simulink library "lib_all"  (Simulink 7.6)
settings: Solver=ode45  SolverMode=SingleTasking  StartTime=0.0  StopTime=10.0  RelTol=1e-3
values from neighbouring files (symbols this model uses):
  map_lib_03 = [6.8679 7.0159 7.4571 8.1849 9.1892 10.456 11.97 13.71 15.655 17.781 20.063 22.474 ... (803 elements, 73x11)]    [scr_simdata.mat]    (also in scr_simdata_all.mat)
  map_lib_06 = [2.5784 2.6727 2.9588 3.4453 4.1444 5.068 6.226 7.6242 9.2634 11.139 13.24 15.552 ... (803 elements, 73x11)]    [scr_simdata.mat]    (also in scr_simdata_all.mat)
  map_lib_09 = [0.0025784 0.0026727 0.0029588 0.0034453 0.0041444 0.005068 0.006226 0.0076242 0.0092634 0.011139 0.01324 0.015552 ... (803 elements, 73x11)]    [scr_simdata.mat]    (also in scr_simdata_all.mat)
  map_lib_10 = [2.74 2.75 2.76 2.77 2.78 2.79 2.8 2.81 2.82 2.83 2.84 2.85 ... (81 elements, 1x81)]    [scr_simdata.mat]    (also in scr_simdata_all.mat)
  map_lib_11 = [1.1983 1.2027 1.207 1.2113 1.2156 1.2198 1.2241 1.2283 1.2326 1.2368 1.241 1.2451 ... (81 elements, 1x81)]    [scr_simdata.mat]    (also in scr_simdata_all.mat)
  map_lib_12 = [2.74 2.75 2.76 2.77 2.78 2.79 2.8 2.81 2.82 2.83 2.84 2.85 ... (81 elements, 1x81)]    [scr_simdata.mat]    (also in scr_simdata_all.mat)
  map_lib_13 = [1.74 1.75 1.76 1.77 1.78 1.79 1.8 1.81 1.82 1.83 1.84 1.85 ... (81 elements, 1x81)]    [scr_simdata.mat]    (also in scr_simdata_all.mat)
  map_lib_16 = [0.00048828125 1.6159 1.8962 2.0821 2.2251 2.3426 2.4433 2.5318 2.611 2.683 2.749 2.8101 ... (1419 elements, 129x11)]    [scr_simdata.mat]    (also in scr_simdata_all.mat)
  map_mr2_01 = [0 800 1600 2400 3200 4000]    [scr_simdata.mat]    (also in scr_simdata_all.mat)
  map_mr2_02 = [1.32 1.32 1.33 1.34 1.36 1.38]    [scr_simdata.mat]    (also in scr_simdata_all.mat)
  map_mr2_03 = [0 800 1600 2400 3200 4000]    [scr_simdata.mat]    (also in scr_simdata_all.mat)
  map_mr2_04 = [1.32 1.32 1.33 1.34 1.36 1.38]    [scr_simdata.mat]    (also in scr_simdata_all.mat)
  map_mr2_05 = [0 800 1600 2400 3200 4000]    [scr_simdata.mat]    (also in scr_simdata_all.mat)
  map_mr2_06 = [1.32 1.32 1.33 1.34 1.36 1.38]    [scr_simdata.mat]    (also in scr_simdata_all.mat)
  Parameter1 = 6    [scr_simdata.mat]    (also in scr_simdata_all.mat)
  Parameter101 = 30    [scr_simdata.mat]    (also in scr_simdata_all.mat)
  Parameter102 = 12    [scr_simdata.mat]    (also in scr_simdata_all.mat)
  Parameter103 = -240    [scr_simdata.mat]    (also in scr_simdata_all.mat)
  Parameter108 = 48    [scr_simdata.mat]    (also in scr_simdata_all.mat)
  Parameter120 = 16    [scr_simdata.mat]    (also in scr_simdata_all.mat)
  Parameter121 = 87    [scr_simdata.mat]    (also in scr_simdata_all.mat)
  Parameter123 = 78    [scr_simdata.mat]    (also in scr_simdata_all.mat)
  Parameter124 = 60    [scr_simdata.mat]    (also in scr_simdata_all.mat)
  Parameter125 = -480    [scr_simdata.mat]    (also in scr_simdata_all.mat)
  Parameter130 = 96    [scr_simdata.mat]    (also in scr_simdata_all.mat)
  Parameter131 = 3    [scr_simdata.mat]    (also in scr_simdata_all.mat)
  Parameter144 = 3    [scr_simdata.mat]    (also in scr_simdata_all.mat)
  Parameter18 = 128    [scr_simdata.mat]    (also in scr_simdata_all.mat)
  Parameter19 = 16    [scr_simdata.mat]    (also in scr_simdata_all.mat)
  Parameter2 = 12    [scr_simdata.mat]    (also in scr_simdata_all.mat)
  Parameter20 = 7    [scr_simdata.mat]    (also in scr_simdata_all.mat)
  Parameter21 = 4    [scr_simdata.mat]    (also in scr_simdata_all.mat)
  Parameter3 = 18    [scr_simdata.mat]    (also in scr_simdata_all.mat)
  Parameter32 = 1    [scr_simdata.mat]    (also in scr_simdata_all.mat)
  Parameter34 = 24    [scr_simdata.mat]    (also in scr_simdata_all.mat)
  Parameter4 = 138    [scr_simdata.mat]    (also in scr_simdata_all.mat)
  Parameter42 = 48    [scr_simdata.mat]    (also in scr_simdata_all.mat)
  Parameter5 = 135    [scr_simdata.mat]    (also in scr_simdata_all.mat)
  Parameter55 = 0    [scr_simdata.mat]    (also in scr_simdata_all.mat)
  Parameter56 = 0    [scr_simdata.mat]    (also in scr_simdata_all.mat)
  Parameter57 = 1    [scr_simdata.mat]    (also in scr_simdata_all.mat)
  Parameter58 = 1    [scr_simdata.mat]    (also in scr_simdata_all.mat)
  Parameter59 = 2    [scr_simdata.mat]    (also in scr_simdata_all.mat)
  Parameter6 = 132    [scr_simdata.mat]    (also in scr_simdata_all.mat)
  Parameter60 = 2    [scr_simdata.mat]    (also in scr_simdata_all.mat)
  Parameter63 = 144    [scr_simdata.mat]    (also in scr_simdata_all.mat)
  Parameter7 = 126    [scr_simdata.mat]    (also in scr_simdata_all.mat)
  Parameter76 = 64    [scr_simdata.mat]    (also in scr_simdata_all.mat)
  Parameter77 = 135    [scr_simdata.mat]    (also in scr_simdata_all.mat)
  Parameter79 = 126    [scr_simdata.mat]    (also in scr_simdata_all.mat)
  Parameter80 = 108    [scr_simdata.mat]    (also in scr_simdata_all.mat)
  Parameter81 = 0    [scr_simdata.mat]    (also in scr_simdata_all.mat)
  Parameter86 = 0    [scr_simdata.mat]    (also in scr_simdata_all.mat)
  Parameter98 = 112    [scr_simdata.mat]    (also in scr_simdata_all.mat)
  Parameter99 = 39    [scr_simdata.mat]    (also in scr_simdata_all.mat)
undefined symbols (not defined in the file or in its folder): cylvolkp_hih_map2h, cylvolkp_map2h, pk1k_map2h
libraries: simulink x2
(part 11 of 36)

== system lib_all/SubSystem1196/SubSystem1273/SubSystem1328/SubSystem1330 ==
  note: Text255
  Block3578 : Inport
  Block3579 : Inport  Port=2
  Block3580 : Inport  Port=3
  Block3581 : Constant  Value=0  OutDataTypeStr=double  SampleTime=-1
  Block3582 : Constant  Value=720  OutDataTypeStr=double  SampleTime=-1
  Block3583 : Gain  Gain=5  SaturateOnIntegerOverflow=on  OutDataTypeStr=Inherit: Same as input  ParamDataTypeStr=Inherit: Same as input
      settings: AttributesFormatString=Attribute703
  Block3584 : Sum  Inputs=+-  OutDataTypeStr=double  RndMeth=Zero  SaturateOnIntegerOverflow=on  InputSameDT=on
  SubSystem1331 : Subsystem
      settings: RTWFcnNameOpts=Use subsystem name
  SubSystem1336 : Subsystem
      settings: RTWSystemCode=Inline
  Block3610 : Outport
  wires:
  Block3583/1 -> Block3584/1
  SubSystem1331/1(Block3603) -> Block3583/1
  Block3578/1 -> SubSystem1331/1(Block3585)
  Block3579/1 -> SubSystem1331/2(Block3586)
  Block3580/1 -> Block3584/2
  Block3584/1 -> SubSystem1336/2(Block3605)
  Block3582/1 -> SubSystem1336/1(Block3604)
  Block3581/1 -> SubSystem1336/3(Block3606)
  SubSystem1336/1(Block3609) -> Block3610/1

== system lib_all/SubSystem1196/SubSystem1273/SubSystem1328/SubSystem1338 ==
  note: Text256
  Block3611 : Inport
  Block3612 : Inport  Port=2
  Block3613 : Constant  Value=360  OutDataTypeStr=double  SampleTime=-1
  Block3614 : Constant  Value=360  OutDataTypeStr=double  SampleTime=-1
  Block3615 : Sum  Inputs=+-  OutDataTypeStr=double  RndMeth=Zero  SaturateOnIntegerOverflow=on  InputSameDT=on
  Block3616 : Switch  Threshold=0.5  RndMeth=Zero  SaturateOnIntegerOverflow=on  InputSameDT=on
  Block3617 : Lookup2D  RowIndex=map_lib_08  ColumnIndex=map_lib_07  Table=map_lib_09  SaturateOnIntegerOverflow=on  InputSameDT=on
      settings: AttributesFormatString=<%<OutputValues>>\nAttribute712
  SubSystem1339 : Subsystem
  Block3618 : Outport
  wires:
  Block3612/1 -> SubSystem1339/1(In1), Block3616/3, Block3615/1
  Block3614/1 -> SubSystem1339/2(In2)
  SubSystem1339/1(Out1) -> Block3616/2
  Block3615/1 -> Block3616/1
  Block3613/1 -> Block3615/2
  Block3617/1 -> Block3618/1
  Block3611/1 -> Block3617/2
  Block3616/1 -> Block3617/1  "SigLabel117"

== system lib_all/SubSystem1196/SubSystem1340/SubSystem1342/SubSystem1343 ==
  Block3632 : Inport
  Block3633 : Inport  Port=2
  Block3634 : Constant  Value=Parameter32  OutDataTypeStr=double  SampleTime=-1
  Block3635 : Constant  Value=2  OutDataTypeStr=double  SampleTime=-1
  Block3636 : Constant  Value=Parameter42  OutDataTypeStr=double  SampleTime=-1
  Block3637 : Constant  Value=0  OutDataTypeStr=double  SampleTime=-1
  Block3638 : Constant  Value=72  OutDataTypeStr=double  SampleTime=-1
  Block3639 : Constant  Value=Parameter34  OutDataTypeStr=double  SampleTime=-1
  Block3640 : Logic  Operator=OR
  Block3641 : Sum  Inputs=+-  OutDataTypeStr=double  RndMeth=Zero  SaturateOnIntegerOverflow=on  InputSameDT=on
  Block3642 : Switch  Threshold=0.5  RndMeth=Zero  SaturateOnIntegerOverflow=on  InputSameDT=on
  Block3643 : Switch  Threshold=0.5  RndMeth=Zero  SaturateOnIntegerOverflow=on  InputSameDT=on
  SubSystem1344 : Subsystem
  SubSystem1345 : Subsystem
  SubSystem1346 : Subsystem
      settings: RTWSystemCode=Inline
  Block3650 : Outport
  wires:
  Block3643/1 -> Block3650/1
  SubSystem1346/1(Block3649) -> Block3643/1
  Block3638/1 -> SubSystem1346/1(Block3644)
  Block3637/1 -> SubSystem1346/3(Block3646)
  Block3641/1 -> SubSystem1346/2(Block3645)
  Block3632/1 -> Block3643/3, Block3641/1
  Block3633/1 -> SubSystem1344/2(In2), SubSystem1345/1(In1)
  Block3634/1 -> SubSystem1344/1(In1)
  Block3635/1 -> SubSystem1345/2(In2)
  SubSystem1344/1(Out1) -> Block3640/1, Block3642/2
  SubSystem1345/1(Out1) -> Block3640/2
  Block3640/1 -> Block3643/2
  Block3636/1 -> Block3642/1
  Block3639/1 -> Block3642/3
  Block3642/1 -> Block3641/2

== system lib_all/SubSystem1196/SubSystem1340/SubSystem1342/SubSystem1348 ==
  Block3651 : Inport
  Block3652 : Inport  Port=2
  Block3653 : Inport  Port=3
  Block3654 : Sum  OutDataTypeStr=double  RndMeth=Zero  SaturateOnIntegerOverflow=on  InputSameDT=on
  Block3655 : Switch  Threshold=0.5  RndMeth=Zero  SaturateOnIntegerOverflow=on  InputSameDT=on
  SubSystem1349 : Subsystem
  Block3656 : Outport
  wires:
  Block3652/1 -> SubSystem1349/1(In1), Block3655/3, Block3654/2
  Block3653/1 -> SubSystem1349/2(In2)
  SubSystem1349/1(Out1) -> Block3655/2
  Block3654/1 -> Block3655/1
  Block3655/1 -> Block3656/1
  Block3651/1 -> Block3654/1

== system lib_all/SubSystem1196/SubSystem1340/SubSystem1350/SubSystem1351 ==
  note: ***編集禁止***
  In1 : Inport
  In2 : Inport  Port=2
  RO1 : RelationalOperator  Operator=>  InputSameDT=on
  single1 : DataTypeConversion  OutDataTypeStr=single  SaturateOnIntegerOverflow=on  RndMeth=Zero
  single2 : DataTypeConversion  OutDataTypeStr=single  SaturateOnIntegerOverflow=on  RndMeth=Zero
  Out1 : Outport
  wires:
  single2/1 -> RO1/2
  single1/1 -> RO1/1
  In1/1 -> single1/1
  In2/1 -> single2/1
  RO1/1 -> Out1/1

== system lib_all/SubSystem1196/SubSystem1352/SubSystem1354/SubSystem1355 ==
  Block3678 : Inport
  Block3679 : Inport  Port=2
  Block3680 : Constant  Value=Parameter32  OutDataTypeStr=double  SampleTime=-1
  Block3681 : Constant  Value=2  OutDataTypeStr=double  SampleTime=-1
  Block3682 : Constant  Value=Parameter42  OutDataTypeStr=double  SampleTime=-1
  Block3683 : Constant  Value=0  OutDataTypeStr=double  SampleTime=-1
  Block3684 : Constant  Value=72  OutDataTypeStr=double  SampleTime=-1
  Block3685 : Constant  Value=Parameter34  OutDataTypeStr=double  SampleTime=-1
  Block3686 : Logic  Operator=OR
  Block3687 : Sum  Inputs=+-  OutDataTypeStr=double  RndMeth=Zero  SaturateOnIntegerOverflow=on  InputSameDT=on
  Block3688 : Switch  Threshold=0.5  RndMeth=Zero  SaturateOnIntegerOverflow=on  InputSameDT=on
  Block3689 : Switch  Threshold=0.5  RndMeth=Zero  SaturateOnIntegerOverflow=on  InputSameDT=on
  SubSystem1356 : Subsystem
  SubSystem1357 : Subsystem
  SubSystem1358 : Subsystem
      settings: RTWSystemCode=Inline
  Block3696 : Outport
  wires:
  Block3689/1 -> Block3696/1
  SubSystem1358/1(Block3695) -> Block3689/1
  Block3684/1 -> SubSystem1358/1(Block3690)
  Block3683/1 -> SubSystem1358/3(Block3692)
  Block3687/1 -> SubSystem1358/2(Block3691)
  Block3678/1 -> Block3689/3, Block3687/1
  Block3679/1 -> SubSystem1356/2(In2), SubSystem1357/1(In1)
  Block3680/1 -> SubSystem1356/1(In1)
  Block3681/1 -> SubSystem1357/2(In2)
  SubSystem1356/1(Out1) -> Block3686/1, Block3688/2
  SubSystem1357/1(Out1) -> Block3686/2
  Block3686/1 -> Block3689/2
  Block3682/1 -> Block3688/1
  Block3685/1 -> Block3688/3
  Block3688/1 -> Block3687/2

== system lib_all/SubSystem1196/SubSystem1352/SubSystem1354/SubSystem1360 ==
  Block3697 : Inport
  Block3698 : Inport  Port=2
  Block3699 : Inport  Port=3
  Block3700 : Sum  OutDataTypeStr=double  RndMeth=Zero  SaturateOnIntegerOverflow=on  InputSameDT=on
  Block3701 : Switch  Threshold=0.5  RndMeth=Zero  SaturateOnIntegerOverflow=on  InputSameDT=on
  SubSystem1361 : Subsystem
  Block3702 : Outport
  wires:
  Block3698/1 -> SubSystem1361/1(In1), Block3701/3, Block3700/2
  Block3699/1 -> SubSystem1361/2(In2)
  SubSystem1361/1(Out1) -> Block3701/2
  Block3700/1 -> Block3701/1
  Block3701/1 -> Block3702/1
  Block3697/1 -> Block3700/1

== system lib_all/SubSystem1196/SubSystem1352/SubSystem1362/SubSystem1363 ==
  note: ***編集禁止***
  In1 : Inport
  In2 : Inport  Port=2
  RO1 : RelationalOperator  Operator=>  InputSameDT=on
  single1 : DataTypeConversion  OutDataTypeStr=single  SaturateOnIntegerOverflow=on  RndMeth=Zero
  single2 : DataTypeConversion  OutDataTypeStr=single  SaturateOnIntegerOverflow=on  RndMeth=Zero
  Out1 : Outport
  wires:
  single2/1 -> RO1/2
  single1/1 -> RO1/1
  In1/1 -> single1/1
  In2/1 -> single2/1
  RO1/1 -> Out1/1

== system lib_all/SubSystem1364/SubSystem1366/SubSystem1369/SubSystem1370 ==
  note: ***編集禁止***
  mult : Inport
  div : Inport  Port=2
  init : Inport  Port=3
  Product : Subsystem  TreatAsAtomicUnit=on
      settings: RTWSystemCode=Inline
  Out : Outport
  wires:
  init/1 -> Product/3(init)
  div/1 -> Product/2(div)
  mult/1 -> Product/1(mult)
  Product/1(Out) -> Out/1

== system lib_all/SubSystem1364/SubSystem1366/SubSystem1369/SubSystem1371 ==
  note: ***編集禁止***
  element : Inport
  Inside_DSR : DataStoreRead  DataStoreName=DSM95
  Selector : Selector  IndexOptions=Index vector (port)  Indices=[1 3]  OutputSizes=1
  Output : Outport
  wires:
  Inside_DSR/1 -> Selector/1
  element/1 -> Selector/2
  Selector/1 -> Output/1

== system lib_all/SubSystem1364/SubSystem1366/SubSystem1369/SubSystem1372 ==
  Block3761 : Inport
  Block3762 : Inport  Port=2
  Block3763 : Inport  Port=3
  Block3764 : Sum  OutDataTypeStr=double  RndMeth=Zero  SaturateOnIntegerOverflow=on  InputSameDT=on
  Block3765 : Sum  Inputs=+-  OutDataTypeStr=double  RndMeth=Zero  SaturateOnIntegerOverflow=on  InputSameDT=on
  Block3766 : Switch  Threshold=0.5  RndMeth=Zero  SaturateOnIntegerOverflow=on  InputSameDT=on
  Block3767 : Switch  Threshold=0.5  RndMeth=Zero  SaturateOnIntegerOverflow=on  InputSameDT=on
  SubSystem1373 : Subsystem
  SubSystem1374 : Subsystem
  Block3768 : Outport
  wires:
  Block3765/1 -> Block3766/1
  Block3766/1 -> Block3768/1
  Block3764/1 -> Block3767/1
  Block3767/1 -> Block3766/3
  SubSystem1374/1(Out1) -> Block3767/2
  Block3763/1 -> SubSystem1374/2(In2)
  SubSystem1373/1(Out1) -> Block3766/2
  Block3761/1 -> SubSystem1373/2(In2), Block3765/2, Block3764/1
  Block3762/1 -> Block3764/2, Block3767/3, SubSystem1374/1(In1), Block3765/1, SubSystem1373/1(In1)

== system lib_all/SubSystem1364/SubSystem1366/SubSystem1375/SubSystem1376 ==
  note: ***編集禁止***
  In1 : Inport
  In2 : Inport  Port=2
  LO1 : Logic
  RO1 : RelationalOperator  Operator=<  InputSameDT=on
  RO2 : RelationalOperator  Operator=~=  InputSameDT=on
  single1 : DataTypeConversion  OutDataTypeStr=single  SaturateOnIntegerOverflow=on  RndMeth=Zero
  single2 : DataTypeConversion  OutDataTypeStr=single  SaturateOnIntegerOverflow=on  RndMeth=Zero
  Out1 : Outport
  wires:
  single2/1 -> RO1/2, RO2/2
  single1/1 -> RO1/1, RO2/1
  In1/1 -> single1/1
  In2/1 -> single2/1
  RO1/1 -> LO1/1
  RO2/1 -> LO1/2
  LO1/1 -> Out1/1

== system lib_all/SubSystem1364/SubSystem1366/SubSystem1375/SubSystem1377 ==
  note: ***編集禁止***
  In1 : Inport
  In2 : Inport  Port=2
  RO1 : RelationalOperator  Operator===  InputSameDT=on
  single1 : DataTypeConversion  OutDataTypeStr=single  SaturateOnIntegerOverflow=on  RndMeth=Zero
  single2 : DataTypeConversion  OutDataTypeStr=single  SaturateOnIntegerOverflow=on  RndMeth=Zero
  Out1 : Outport
  wires:
  single2/1 -> RO1/2
  single1/1 -> RO1/1
  In1/1 -> single1/1
  In2/1 -> single2/1
  RO1/1 -> Out1/1

== system lib_all/SubSystem1364/SubSystem1366/SubSystem1375/SubSystem1378 ==
  note: ***編集禁止***
  In1 : Inport
  In2 : Inport  Port=2
  RO1 : RelationalOperator  Operator===  InputSameDT=on
  single1 : DataTypeConversion  OutDataTypeStr=single  SaturateOnIntegerOverflow=on  RndMeth=Zero
  single2 : DataTypeConversion  OutDataTypeStr=single  SaturateOnIntegerOverflow=on  RndMeth=Zero
  Out1 : Outport
  wires:
  single2/1 -> RO1/2
  single1/1 -> RO1/1
  In1/1 -> single1/1
  In2/1 -> single2/1
  RO1/1 -> Out1/1

== system lib_all/SubSystem1364/SubSystem1366/SubSystem1375/SubSystem1379 ==
  note: ***編集禁止***
  In1 : Inport
  In2 : Inport  Port=2
  RO1 : RelationalOperator  Operator===  InputSameDT=on
  single1 : DataTypeConversion  OutDataTypeStr=single  SaturateOnIntegerOverflow=on  RndMeth=Zero
  single2 : DataTypeConversion  OutDataTypeStr=single  SaturateOnIntegerOverflow=on  RndMeth=Zero
  Out1 : Outport
  wires:
  single2/1 -> RO1/2
  single1/1 -> RO1/1
  In1/1 -> single1/1
  In2/1 -> single2/1
  RO1/1 -> Out1/1

== system lib_all/SubSystem1364/SubSystem1366/SubSystem1375/SubSystem1380 ==
  Block3791 : Inport
  Block3792 : Inport  Port=2
  Block3793 : EnablePort
  Block3794 : Demux  Outputs=2
  Block3795 : Mux  Inputs=2
  SubSystem1381 : Subsystem
  SubSystem1385 : Subsystem
  Block3804 : Outport
  wires:
  Block3795/1 -> Block3804/1
  SubSystem1385/1(Block3803) -> Block3795/2
  SubSystem1381/1(Block3799) -> Block3795/1
  Block3794/2 -> SubSystem1385/2(Block3801)
  Block3794/1 -> SubSystem1381/2(Block3797)
  Block3792/1 -> SubSystem1381/1(Block3796), SubSystem1385/1(Block3800)
  Block3791/1 -> Block3794/1

== system lib_all/SubSystem1364/SubSystem1366/SubSystem1375/SubSystem1389 ==
  Block3805 : Inport
  Block3806 : Inport  Port=2
  Block3807 : EnablePort
  Block3808 : Demux  Outputs=2
  Block3809 : Mux  Inputs=2
  Block3810 : Sum  OutDataTypeStr=double  RndMeth=Zero  SaturateOnIntegerOverflow=on  InputSameDT=on
  SubSystem1390 : Subsystem  TreatAsAtomicUnit=on  mask(***ATC.DSRVEC***){bd_version=1.01.A, r0=DSM1, r1=-1, r2=, r3=, r4=, r5=, r6=, r7=, r8=, r9=, c0=, c1=, c2=, c3=, c4=, c5=, c6=, c7=, c8=, c9=}
      mask prompts: bd_version "Version"
      mask help: これはAutoCからのブロックです．編集禁止．
      settings: OpenFcn=warndlg('変更禁止!');
      mask Help: web(ui_blockhelpmng(gcb));
      mask Display: disp('ATC.DSRVEC')
  SubSystem1391 : Subsystem
  SubSystem1395 : Subsystem
  Block3819 : Outport
  wires:
  Block3806/1 -> SubSystem1390/1(element), SubSystem1391/1(Block3811), SubSystem1395/1(Block3815)
  Block3809/1 -> Block3810/2
  SubSystem1390/1(Output) -> Block3810/1
  Block3810/1 -> Block3819/1
  SubSystem1395/1(Block3818) -> Block3809/2
  SubSystem1391/1(Block3814) -> Block3809/1
  Block3808/2 -> SubSystem1395/2(Block3816)
  Block3808/1 -> SubSystem1391/2(Block3812)
  Block3805/1 -> Block3808/1

== system lib_all/SubSystem1364/SubSystem1366/SubSystem1375/SubSystem1399 ==
  Block3820 : Inport
  Block3821 : Inport  Port=2
  Block3822 : EnablePort
  Block3823 : Demux  Outputs=2
  Block3824 : Mux  Inputs=2
  Block3825 : Sum  OutDataTypeStr=double  RndMeth=Zero  SaturateOnIntegerOverflow=on  InputSameDT=on
  SubSystem1400 : Subsystem  TreatAsAtomicUnit=on  mask(***ATC.DSRVEC***){bd_version=1.01.A, r0=DSM5, r1=-1, r2=, r3=, r4=, r5=, r6=, r7=, r8=, r9=, c0=, c1=, c2=, c3=, c4=, c5=, c6=, c7=, c8=, c9=}
      mask prompts: bd_version "Version"
      mask help: これはAutoCからのブロックです．編集禁止．
      settings: OpenFcn=warndlg('変更禁止!');
      mask Help: web(ui_blockhelpmng(gcb));
      mask Display: disp('ATC.DSRVEC')
  SubSystem1401 : Subsystem
  SubSystem1405 : Subsystem
  Block3834 : Outport
  wires:
  Block3821/1 -> SubSystem1400/1(element), SubSystem1401/1(Block3826), SubSystem1405/1(Block3830)
  Block3824/1 -> Block3825/2
  SubSystem1400/1(Output) -> Block3825/1
  Block3825/1 -> Block3834/1
  SubSystem1405/1(Block3833) -> Block3824/2
  SubSystem1401/1(Block3829) -> Block3824/1
  Block3823/2 -> SubSystem1405/2(Block3831)
  Block3823/1 -> SubSystem1401/2(Block3827)
  Block3820/1 -> Block3823/1

== system lib_all/SubSystem1364/SubSystem1366/SubSystem1375/SubSystem1409 ==
  Block3835 : Inport
  Block3836 : Inport  Port=2
  Block3837 : EnablePort
  Block3838 : Demux  Outputs=2
  Block3839 : Mux  Inputs=2
  Block3840 : Sum  OutDataTypeStr=double  RndMeth=Zero  SaturateOnIntegerOverflow=on  InputSameDT=on
  SubSystem1410 : Subsystem  TreatAsAtomicUnit=on  mask(***ATC.DSRVEC***){bd_version=1.01.A, r0=DSM3, r1=-1, r2=, r3=, r4=, r5=, r6=, r7=, r8=, r9=, c0=, c1=, c2=, c3=, c4=, c5=, c6=, c7=, c8=, c9=}
      mask prompts: bd_version "Version"
      mask help: これはAutoCからのブロックです．編集禁止．
      settings: OpenFcn=warndlg('変更禁止!');
      mask Help: web(ui_blockhelpmng(gcb));
      mask Display: disp('ATC.DSRVEC')
  SubSystem1411 : Subsystem
  SubSystem1415 : Subsystem
  Block3849 : Outport
  wires:
  Block3836/1 -> SubSystem1410/1(element), SubSystem1411/1(Block3841), SubSystem1415/1(Block3845)
  Block3835/1 -> Block3838/1
  Block3838/1 -> SubSystem1411/2(Block3842)
  Block3838/2 -> SubSystem1415/2(Block3846)
  SubSystem1411/1(Block3844) -> Block3839/1
  SubSystem1415/1(Block3848) -> Block3839/2
  Block3840/1 -> Block3849/1
  SubSystem1410/1(Output) -> Block3840/1
  Block3839/1 -> Block3840/2

== system lib_all/SubSystem1364/SubSystem1419/SubSystem1422/SubSystem1423 ==
  note: ***編集禁止***
  mult : Inport
  div : Inport  Port=2
  init : Inport  Port=3
  Product : Subsystem  TreatAsAtomicUnit=on
      settings: RTWSystemCode=Inline
  Out : Outport
  wires:
  init/1 -> Product/3(init)
  div/1 -> Product/2(div)
  mult/1 -> Product/1(mult)
  Product/1(Out) -> Out/1

== system lib_all/SubSystem1364/SubSystem1419/SubSystem1422/SubSystem1424 ==
  note: ***編集禁止***
  element : Inport
  Inside_DSR : DataStoreRead  DataStoreName=DSM95
  Selector : Selector  IndexOptions=Index vector (port)  Indices=[1 3]  OutputSizes=1
  Output : Outport
  wires:
  Inside_DSR/1 -> Selector/1
  element/1 -> Selector/2
  Selector/1 -> Output/1

== system lib_all/SubSystem1364/SubSystem1419/SubSystem1422/SubSystem1425 ==
  Block3883 : Inport
  Block3884 : Inport  Port=2
  Block3885 : Inport  Port=3
  Block3886 : Sum  OutDataTypeStr=double  RndMeth=Zero  SaturateOnIntegerOverflow=on  InputSameDT=on
  Block3887 : Sum  Inputs=+-  OutDataTypeStr=double  RndMeth=Zero  SaturateOnIntegerOverflow=on  InputSameDT=on
  Block3888 : Switch  Threshold=0.5  RndMeth=Zero  SaturateOnIntegerOverflow=on  InputSameDT=on
  Block3889 : Switch  Threshold=0.5  RndMeth=Zero  SaturateOnIntegerOverflow=on  InputSameDT=on
  SubSystem1426 : Subsystem
  SubSystem1427 : Subsystem
  Block3890 : Outport
  wires:
  Block3887/1 -> Block3888/1
  Block3888/1 -> Block3890/1
  Block3886/1 -> Block3889/1
  Block3889/1 -> Block3888/3
  SubSystem1427/1(Out1) -> Block3889/2
  Block3885/1 -> SubSystem1427/2(In2)
  SubSystem1426/1(Out1) -> Block3888/2
  Block3883/1 -> SubSystem1426/2(In2), Block3887/2, Block3886/1
  Block3884/1 -> Block3886/2, Block3889/3, SubSystem1427/1(In1), Block3887/1, SubSystem1426/1(In1)

== system lib_all/SubSystem1364/SubSystem1419/SubSystem1428/SubSystem1429 ==
  note: ***編集禁止***
  In1 : Inport
  In2 : Inport  Port=2
  LO1 : Logic
  RO1 : RelationalOperator  Operator=<  InputSameDT=on
  RO2 : RelationalOperator  Operator=~=  InputSameDT=on
  single1 : DataTypeConversion  OutDataTypeStr=single  SaturateOnIntegerOverflow=on  RndMeth=Zero
  single2 : DataTypeConversion  OutDataTypeStr=single  SaturateOnIntegerOverflow=on  RndMeth=Zero
  Out1 : Outport
  wires:
  single2/1 -> RO1/2, RO2/2
  single1/1 -> RO1/1, RO2/1
  In1/1 -> single1/1
  In2/1 -> single2/1
  RO1/1 -> LO1/1
  RO2/1 -> LO1/2
  LO1/1 -> Out1/1

== system lib_all/SubSystem1364/SubSystem1419/SubSystem1428/SubSystem1430 ==
  note: ***編集禁止***
  In1 : Inport
  In2 : Inport  Port=2
  RO1 : RelationalOperator  Operator===  InputSameDT=on
  single1 : DataTypeConversion  OutDataTypeStr=single  SaturateOnIntegerOverflow=on  RndMeth=Zero
  single2 : DataTypeConversion  OutDataTypeStr=single  SaturateOnIntegerOverflow=on  RndMeth=Zero
  Out1 : Outport
  wires:
  single2/1 -> RO1/2
  single1/1 -> RO1/1
  In1/1 -> single1/1
  In2/1 -> single2/1
  RO1/1 -> Out1/1

== system lib_all/SubSystem1364/SubSystem1419/SubSystem1428/SubSystem1431 ==
  note: ***編集禁止***
  In1 : Inport
  In2 : Inport  Port=2
  RO1 : RelationalOperator  Operator===  InputSameDT=on
  single1 : DataTypeConversion  OutDataTypeStr=single  SaturateOnIntegerOverflow=on  RndMeth=Zero
  single2 : DataTypeConversion  OutDataTypeStr=single  SaturateOnIntegerOverflow=on  RndMeth=Zero
  Out1 : Outport
  wires:
  single2/1 -> RO1/2
  single1/1 -> RO1/1
  In1/1 -> single1/1
  In2/1 -> single2/1
  RO1/1 -> Out1/1

== system lib_all/SubSystem1364/SubSystem1419/SubSystem1428/SubSystem1432 ==
  note: ***編集禁止***
  In1 : Inport
  In2 : Inport  Port=2
  RO1 : RelationalOperator  Operator===  InputSameDT=on
  single1 : DataTypeConversion  OutDataTypeStr=single  SaturateOnIntegerOverflow=on  RndMeth=Zero
  single2 : DataTypeConversion  OutDataTypeStr=single  SaturateOnIntegerOverflow=on  RndMeth=Zero
  Out1 : Outport
  wires:
  single2/1 -> RO1/2
  single1/1 -> RO1/1
  In1/1 -> single1/1
  In2/1 -> single2/1
  RO1/1 -> Out1/1

== system lib_all/SubSystem1364/SubSystem1419/SubSystem1428/SubSystem1433 ==
  Block3913 : Inport
  Block3914 : Inport  Port=2
  Block3915 : EnablePort
  Block3916 : Demux  Outputs=2
  Block3917 : Mux  Inputs=2
  SubSystem1434 : Subsystem
  SubSystem1438 : Subsystem
  Block3926 : Outport
  wires:
  Block3917/1 -> Block3926/1
  SubSystem1438/1(Block3925) -> Block3917/2
  SubSystem1434/1(Block3921) -> Block3917/1
  Block3916/2 -> SubSystem1438/2(Block3923)
  Block3916/1 -> SubSystem1434/2(Block3919)
  Block3914/1 -> SubSystem1434/1(Block3918), SubSystem1438/1(Block3922)
  Block3913/1 -> Block3916/1

== system lib_all/SubSystem1364/SubSystem1419/SubSystem1428/SubSystem1442 ==
  Block3927 : Inport
  Block3928 : Inport  Port=2
  Block3929 : EnablePort
  Block3930 : Demux  Outputs=2
  Block3931 : Mux  Inputs=2
  Block3932 : Sum  OutDataTypeStr=double  RndMeth=Zero  SaturateOnIntegerOverflow=on  InputSameDT=on
  SubSystem1443 : Subsystem  TreatAsAtomicUnit=on  mask(***ATC.DSRVEC***){bd_version=1.01.A, r0=DSM1, r1=-1, r2=, r3=, r4=, r5=, r6=, r7=, r8=, r9=, c0=, c1=, c2=, c3=, c4=, c5=, c6=, c7=, c8=, c9=}
      mask prompts: bd_version "Version"
      mask help: これはAutoCからのブロックです．編集禁止．
      settings: OpenFcn=warndlg('変更禁止!');
      mask Help: web(ui_blockhelpmng(gcb));
      mask Display: disp('ATC.DSRVEC')
  SubSystem1444 : Subsystem
  SubSystem1448 : Subsystem
  Block3941 : Outport
  wires:
  Block3928/1 -> SubSystem1443/1(element), SubSystem1444/1(Block3933), SubSystem1448/1(Block3937)
  Block3931/1 -> Block3932/2
  SubSystem1443/1(Output) -> Block3932/1
  Block3932/1 -> Block3941/1
  SubSystem1448/1(Block3940) -> Block3931/2
  SubSystem1444/1(Block3936) -> Block3931/1
  Block3930/2 -> SubSystem1448/2(Block3938)
  Block3930/1 -> SubSystem1444/2(Block3934)
  Block3927/1 -> Block3930/1

== system lib_all/SubSystem1364/SubSystem1419/SubSystem1428/SubSystem1452 ==
  Block3942 : Inport
  Block3943 : Inport  Port=2
  Block3944 : EnablePort
  Block3945 : Demux  Outputs=2
  Block3946 : Mux  Inputs=2
  Block3947 : Sum  OutDataTypeStr=double  RndMeth=Zero  SaturateOnIntegerOverflow=on  InputSameDT=on
  SubSystem1453 : Subsystem  TreatAsAtomicUnit=on  mask(***ATC.DSRVEC***){bd_version=1.01.A, r0=DSM5, r1=-1, r2=, r3=, r4=, r5=, r6=, r7=, r8=, r9=, c0=, c1=, c2=, c3=, c4=, c5=, c6=, c7=, c8=, c9=}
      mask prompts: bd_version "Version"
      mask help: これはAutoCからのブロックです．編集禁止．
      settings: OpenFcn=warndlg('変更禁止!');
      mask Help: web(ui_blockhelpmng(gcb));
      mask Display: disp('ATC.DSRVEC')
  SubSystem1454 : Subsystem
  SubSystem1458 : Subsystem
  Block3956 : Outport
  wires:
  Block3943/1 -> SubSystem1453/1(element), SubSystem1454/1(Block3948), SubSystem1458/1(Block3952)
  Block3946/1 -> Block3947/2
  SubSystem1453/1(Output) -> Block3947/1
  Block3947/1 -> Block3956/1
  SubSystem1458/1(Block3955) -> Block3946/2
  SubSystem1454/1(Block3951) -> Block3946/1
  Block3945/2 -> SubSystem1458/2(Block3953)
  Block3945/1 -> SubSystem1454/2(Block3949)
  Block3942/1 -> Block3945/1

== system lib_all/SubSystem1364/SubSystem1419/SubSystem1428/SubSystem1462 ==
  Block3957 : Inport
  Block3958 : Inport  Port=2
  Block3959 : EnablePort
  Block3960 : Demux  Outputs=2
  Block3961 : Mux  Inputs=2
  Block3962 : Sum  OutDataTypeStr=double  RndMeth=Zero  SaturateOnIntegerOverflow=on  InputSameDT=on
  SubSystem1463 : Subsystem  TreatAsAtomicUnit=on  mask(***ATC.DSRVEC***){bd_version=1.01.A, r0=DSM3, r1=-1, r2=, r3=, r4=, r5=, r6=, r7=, r8=, r9=, c0=, c1=, c2=, c3=, c4=, c5=, c6=, c7=, c8=, c9=}
      mask prompts: bd_version "Version"
      mask help: これはAutoCからのブロックです．編集禁止．
      settings: OpenFcn=warndlg('変更禁止!');
      mask Help: web(ui_blockhelpmng(gcb));
      mask Display: disp('ATC.DSRVEC')
  SubSystem1464 : Subsystem
  SubSystem1468 : Subsystem
  Block3971 : Outport
  wires:
  Block3958/1 -> SubSystem1463/1(element), SubSystem1464/1(Block3963), SubSystem1468/1(Block3967)
  Block3957/1 -> Block3960/1
  Block3960/1 -> SubSystem1464/2(Block3964)
  Block3960/2 -> SubSystem1468/2(Block3968)
  SubSystem1464/1(Block3966) -> Block3961/1
  SubSystem1468/1(Block3970) -> Block3961/2
  Block3962/1 -> Block3971/1
  SubSystem1463/1(Output) -> Block3962/1
  Block3961/1 -> Block3962/2

== system lib_all/SubSystem1487/SubSystem1490/SubSystem1491/Product ==
  note: ***編集禁止***
  mult : Inport
  div : Inport  Port=2
  init : Inport  Port=3
  Constant1 : Constant  OutDataTypeStr=Inherit: Inherit via back propagation
  Constant2 : Constant  Value=0  OutDataTypeStr=Inherit: Inherit via back propagation
  Product : Product  Inputs=*/  SaturateOnIntegerOverflow=on  RndMeth=Zero  InputSameDT=on  OutDataTypeStr=Inherit: Same as first input
  Relational Operator : RelationalOperator  Operator=~=  InputSameDT=on
  Switch : Switch  Threshold=0.5  SaturateOnIntegerOverflow=on  InputSameDT=on
  Switch1 : Switch  Threshold=0.5  SaturateOnIntegerOverflow=on  InputSameDT=on
  Out : Outport
  wires:
  mult/1 -> Product/1
  Product/1 -> Switch/1
  Relational Operator/1 -> Switch/2, Switch1/2
  init/1 -> Switch/3
  Switch/1 -> Out/1
  div/1 -> Switch1/1, Relational Operator/1
  Switch1/1 -> Product/2
  Constant2/1 -> Relational Operator/2
  Constant1/1 -> Switch1/3

== system lib_all/SubSystem1487/SubSystem1490/SubSystem1493/SubSystem1494 ==
  note: ***編集禁止***
  In1 : Inport
  In2 : Inport  Port=2
  RO1 : RelationalOperator  Operator=>  InputSameDT=on
  single1 : DataTypeConversion  OutDataTypeStr=single  SaturateOnIntegerOverflow=on  RndMeth=Zero
  single2 : DataTypeConversion  OutDataTypeStr=single  SaturateOnIntegerOverflow=on  RndMeth=Zero
  Out1 : Outport
  wires:
  RO1/1 -> Out1/1
  In2/1 -> single2/1
  In1/1 -> single1/1
  single1/1 -> RO1/1
  single2/1 -> RO1/2

== system lib_all/SubSystem1487/SubSystem1490/SubSystem1493/SubSystem1495 ==
  note: ***編集禁止***
  In1 : Inport
  In2 : Inport  Port=2
  LO1 : Logic
  RO1 : RelationalOperator  Operator=<  InputSameDT=on
  RO2 : RelationalOperator  Operator=~=  InputSameDT=on
  single1 : DataTypeConversion  OutDataTypeStr=single  SaturateOnIntegerOverflow=on  RndMeth=Zero
  single2 : DataTypeConversion  OutDataTypeStr=single  SaturateOnIntegerOverflow=on  RndMeth=Zero
  Out1 : Outport
  wires:
  In2/1 -> single2/1
  In1/1 -> single1/1
  single1/1 -> RO1/1, RO2/1
  single2/1 -> RO1/2, RO2/2
  RO1/1 -> LO1/1
  RO2/1 -> LO1/2
  LO1/1 -> Out1/1

== system lib_all/SubSystem1487/SubSystem1496/SubSystem1501/SubSystem1502 ==
  Block4085 : Inport
  Block4086 : Inport  Port=2
  Block4087 : MultiPortSwitch  zeroidx=off  RndMeth=Zero  SaturateOnIntegerOverflow=on  InputSameDT=on
  SubSystem1503 : Subsystem  TreatAsAtomicUnit=on  mask(***ATC.DSRVEC***){bd_version=1.01.A, r0=DSM7, r1=-1, r2=, r3=, r4=, r5=, r6=, r7=, r8=, r9=, c0=, c1=, c2=, c3=, c4=, c5=, c6=, c7=, c8=, c9=}
      mask prompts: bd_version "Version"
      mask help: これはAutoCからのブロックです．編集禁止．
      settings: OpenFcn=warndlg('変更禁止!');
      mask Help: web(ui_blockhelpmng(gcb));
      mask Display: disp('ATC.DSRVEC')
  SubSystem1504 : Subsystem  TreatAsAtomicUnit=on  mask(***ATC.DSRVEC***){bd_version=1.01.A, r0=DSM6, r1=-1, r2=, r3=, r4=, r5=, r6=, r7=, r8=, r9=, c0=, c1=, c2=, c3=, c4=, c5=, c6=, c7=, c8=, c9=}
      mask prompts: bd_version "Version"
      mask help: これはAutoCからのブロックです．編集禁止．
      settings: OpenFcn=warndlg('変更禁止!');
      mask Help: web(ui_blockhelpmng(gcb));
      mask Display: disp('ATC.DSRVEC')
  SubSystem1505 : Subsystem  TreatAsAtomicUnit=on  mask(***ATC.DSRVEC***){bd_version=1.01.A, r0=DSM19, r1=-1, r2=, r3=, r4=, r5=, r6=, r7=, r8=, r9=, c0=, c1=, c2=, c3=, c4=, c5=, c6=, c7=, c8=, c9=}
      mask prompts: bd_version "Version"
      mask help: これはAutoCからのブロックです．編集禁止．
      settings: OpenFcn=warndlg('変更禁止!');
      mask Help: web(ui_blockhelpmng(gcb));
      mask Display: disp('ATC.DSRVEC')
  Block4088 : Outport
  wires:
  Block4085/1 -> Block4087/1
  Block4087/1 -> Block4088/1
  SubSystem1503/1(Output) -> Block4087/2
  SubSystem1504/1(Output) -> Block4087/3
  SubSystem1505/1(Output) -> Block4087/4
  Block4086/1 -> SubSystem1503/1(element), SubSystem1504/1(element), SubSystem1505/1(element)

== system lib_all/SubSystem1487/SubSystem1496/SubSystem1501/SubSystem1506 ==
  Block4089 : Inport
  Block4090 : Inport  Port=2
  Block4091 : MultiPortSwitch  zeroidx=off  RndMeth=Zero  SaturateOnIntegerOverflow=on  InputSameDT=on
  SubSystem1507 : Subsystem  TreatAsAtomicUnit=on  mask(***ATC.DSRVEC***){bd_version=1.01.A, r0=DSM7, r1=-1, r2=, r3=, r4=, r5=, r6=, r7=, r8=, r9=, c0=, c1=, c2=, c3=, c4=, c5=, c6=, c7=, c8=, c9=}
      mask prompts: bd_version "Version"
      mask help: これはAutoCからのブロックです．編集禁止．
      settings: OpenFcn=warndlg('変更禁止!');
      mask Help: web(ui_blockhelpmng(gcb));
      mask Display: disp('ATC.DSRVEC')
  SubSystem1508 : Subsystem  TreatAsAtomicUnit=on  mask(***ATC.DSRVEC***){bd_version=1.01.A, r0=DSM6, r1=-1, r2=, r3=, r4=, r5=, r6=, r7=, r8=, r9=, c0=, c1=, c2=, c3=, c4=, c5=, c6=, c7=, c8=, c9=}
      mask prompts: bd_version "Version"
      mask help: これはAutoCからのブロックです．編集禁止．
      settings: OpenFcn=warndlg('変更禁止!');
      mask Help: web(ui_blockhelpmng(gcb));
      mask Display: disp('ATC.DSRVEC')
  SubSystem1509 : Subsystem  TreatAsAtomicUnit=on  mask(***ATC.DSRVEC***){bd_version=1.01.A, r0=DSM19, r1=-1, r2=, r3=, r4=, r5=, r6=, r7=, r8=, r9=, c0=, c1=, c2=, c3=, c4=, c5=, c6=, c7=, c8=, c9=}
      mask prompts: bd_version "Version"
      mask help: これはAutoCからのブロックです．編集禁止．
      settings: OpenFcn=warndlg('変更禁止!');
      mask Help: web(ui_blockhelpmng(gcb));
      mask Display: disp('ATC.DSRVEC')
  Block4092 : Outport
  wires:
  Block4089/1 -> Block4091/1
  Block4091/1 -> Block4092/1
  SubSystem1507/1(Output) -> Block4091/2
  SubSystem1508/1(Output) -> Block4091/3
  SubSystem1509/1(Output) -> Block4091/4
  Block4090/1 -> SubSystem1507/1(element), SubSystem1508/1(element), SubSystem1509/1(element)

== system lib_all/SubSystem1487/SubSystem1496/SubSystem1510/SubSystem1511 ==
  note: ***編集禁止***
  element : Inport
  Inside_DSR : DataStoreRead  DataStoreName=DSM1
  Selector : Selector  IndexOptions=Index vector (port)  Indices=[1 3]  OutputSizes=1
  Output : Outport
  wires:
  Selector/1 -> Output/1
  element/1 -> Selector/2
  Inside_DSR/1 -> Selector/1

== system lib_all/SubSystem1487/SubSystem1496/SubSystem1510/SubSystem1512 ==
  Block4100 : Inport
  Block4101 : Inport  Port=2
  Block4102 : MultiPortSwitch  zeroidx=off  RndMeth=Zero  SaturateOnIntegerOverflow=on  InputSameDT=on
  SubSystem1513 : Subsystem  TreatAsAtomicUnit=on  mask(***ATC.DSRVEC***){bd_version=1.01.A, r0=DSM7, r1=-1, r2=, r3=, r4=, r5=, r6=, r7=, r8=, r9=, c0=, c1=, c2=, c3=, c4=, c5=, c6=, c7=, c8=, c9=}
      mask prompts: bd_version "Version"
      mask help: これはAutoCからのブロックです．編集禁止．
      settings: OpenFcn=warndlg('変更禁止!');
      mask Help: web(ui_blockhelpmng(gcb));
      mask Display: disp('ATC.DSRVEC')
  SubSystem1514 : Subsystem  TreatAsAtomicUnit=on  mask(***ATC.DSRVEC***){bd_version=1.01.A, r0=DSM6, r1=-1, r2=, r3=, r4=, r5=, r6=, r7=, r8=, r9=, c0=, c1=, c2=, c3=, c4=, c5=, c6=, c7=, c8=, c9=}
      mask prompts: bd_version "Version"
      mask help: これはAutoCからのブロックです．編集禁止．
      settings: OpenFcn=warndlg('変更禁止!');
      mask Help: web(ui_blockhelpmng(gcb));
      mask Display: disp('ATC.DSRVEC')
  SubSystem1515 : Subsystem  TreatAsAtomicUnit=on  mask(***ATC.DSRVEC***){bd_version=1.01.A, r0=DSM19, r1=-1, r2=, r3=, r4=, r5=, r6=, r7=, r8=, r9=, c0=, c1=, c2=, c3=, c4=, c5=, c6=, c7=, c8=, c9=}
      mask prompts: bd_version "Version"
      mask help: これはAutoCからのブロックです．編集禁止．
      settings: OpenFcn=warndlg('変更禁止!');
      mask Help: web(ui_blockhelpmng(gcb));
      mask Display: disp('ATC.DSRVEC')
  Block4103 : Outport
  wires:
  Block4100/1 -> Block4102/1
  Block4102/1 -> Block4103/1
  SubSystem1513/1(Output) -> Block4102/2
  SubSystem1514/1(Output) -> Block4102/3
  SubSystem1515/1(Output) -> Block4102/4
  Block4101/1 -> SubSystem1513/1(element), SubSystem1514/1(element), SubSystem1515/1(element)

== system lib_all/SubSystem1487/SubSystem1496/SubSystem1510/SubSystem1516 ==
  Block4104 : Inport
  Block4105 : Inport  Port=2
  Block4106 : MultiPortSwitch  zeroidx=off  RndMeth=Zero  SaturateOnIntegerOverflow=on  InputSameDT=on
  SubSystem1517 : Subsystem  TreatAsAtomicUnit=on  mask(***ATC.DSRVEC***){bd_version=1.01.A, r0=DSM7, r1=-1, r2=, r3=, r4=, r5=, r6=, r7=, r8=, r9=, c0=, c1=, c2=, c3=, c4=, c5=, c6=, c7=, c8=, c9=}
      mask prompts: bd_version "Version"
      mask help: これはAutoCからのブロックです．編集禁止．
      settings: OpenFcn=warndlg('変更禁止!');
      mask Help: web(ui_blockhelpmng(gcb));
      mask Display: disp('ATC.DSRVEC')
  SubSystem1518 : Subsystem  TreatAsAtomicUnit=on  mask(***ATC.DSRVEC***){bd_version=1.01.A, r0=DSM6, r1=-1, r2=, r3=, r4=, r5=, r6=, r7=, r8=, r9=, c0=, c1=, c2=, c3=, c4=, c5=, c6=, c7=, c8=, c9=}
      mask prompts: bd_version "Version"
      mask help: これはAutoCからのブロックです．編集禁止．
      settings: OpenFcn=warndlg('変更禁止!');
      mask Help: web(ui_blockhelpmng(gcb));
      mask Display: disp('ATC.DSRVEC')
  SubSystem1519 : Subsystem  TreatAsAtomicUnit=on  mask(***ATC.DSRVEC***){bd_version=1.01.A, r0=DSM19, r1=-1, r2=, r3=, r4=, r5=, r6=, r7=, r8=, r9=, c0=, c1=, c2=, c3=, c4=, c5=, c6=, c7=, c8=, c9=}
      mask prompts: bd_version "Version"
      mask help: これはAutoCからのブロックです．編集禁止．
      settings: OpenFcn=warndlg('変更禁止!');
      mask Help: web(ui_blockhelpmng(gcb));
      mask Display: disp('ATC.DSRVEC')
  Block4107 : Outport
  wires:
  Block4104/1 -> Block4106/1
  Block4106/1 -> Block4107/1
  SubSystem1517/1(Output) -> Block4106/2
  SubSystem1518/1(Output) -> Block4106/3
  SubSystem1519/1(Output) -> Block4106/4
  Block4105/1 -> SubSystem1517/1(element), SubSystem1518/1(element), SubSystem1519/1(element)

== system lib_all/SubSystem1487/SubSystem1496/SubSystem1520/SubSystem1521 ==
  note: ***編集禁止***
  element : Inport
  Inside_DSR : DataStoreRead  DataStoreName=DSM5
  Selector : Selector  IndexOptions=Index vector (port)  Indices=[1 3]  OutputSizes=1
  Output : Outport
  wires:
  Selector/1 -> Output/1
  element/1 -> Selector/2
  Inside_DSR/1 -> Selector/1

== system lib_all/SubSystem1487/SubSystem1496/SubSystem1520/SubSystem1522 ==
  Block4115 : Inport
  Block4116 : Inport  Port=2
  Block4117 : MultiPortSwitch  zeroidx=off  RndMeth=Zero  SaturateOnIntegerOverflow=on  InputSameDT=on
  SubSystem1523 : Subsystem  TreatAsAtomicUnit=on  mask(***ATC.DSRVEC***){bd_version=1.01.A, r0=DSM7, r1=-1, r2=, r3=, r4=, r5=, r6=, r7=, r8=, r9=, c0=, c1=, c2=, c3=, c4=, c5=, c6=, c7=, c8=, c9=}
      mask prompts: bd_version "Version"
      mask help: これはAutoCからのブロックです．編集禁止．
      settings: OpenFcn=warndlg('変更禁止!');
      mask Help: web(ui_blockhelpmng(gcb));
      mask Display: disp('ATC.DSRVEC')
  SubSystem1524 : Subsystem  TreatAsAtomicUnit=on  mask(***ATC.DSRVEC***){bd_version=1.01.A, r0=DSM6, r1=-1, r2=, r3=, r4=, r5=, r6=, r7=, r8=, r9=, c0=, c1=, c2=, c3=, c4=, c5=, c6=, c7=, c8=, c9=}
      mask prompts: bd_version "Version"
      mask help: これはAutoCからのブロックです．編集禁止．
      settings: OpenFcn=warndlg('変更禁止!');
      mask Help: web(ui_blockhelpmng(gcb));
      mask Display: disp('ATC.DSRVEC')
  SubSystem1525 : Subsystem  TreatAsAtomicUnit=on  mask(***ATC.DSRVEC***){bd_version=1.01.A, r0=DSM19, r1=-1, r2=, r3=, r4=, r5=, r6=, r7=, r8=, r9=, c0=, c1=, c2=, c3=, c4=, c5=, c6=, c7=, c8=, c9=}
      mask prompts: bd_version "Version"
      mask help: これはAutoCからのブロックです．編集禁止．
      settings: OpenFcn=warndlg('変更禁止!');
      mask Help: web(ui_blockhelpmng(gcb));
      mask Display: disp('ATC.DSRVEC')
  Block4118 : Outport
  wires:
  Block4115/1 -> Block4117/1
  Block4117/1 -> Block4118/1
  SubSystem1523/1(Output) -> Block4117/2
  SubSystem1524/1(Output) -> Block4117/3
  SubSystem1525/1(Output) -> Block4117/4
  Block4116/1 -> SubSystem1523/1(element), SubSystem1524/1(element), SubSystem1525/1(element)

== system lib_all/SubSystem1487/SubSystem1496/SubSystem1520/SubSystem1526 ==
  Block4119 : Inport
  Block4120 : Inport  Port=2
  Block4121 : MultiPortSwitch  zeroidx=off  RndMeth=Zero  SaturateOnIntegerOverflow=on  InputSameDT=on
  SubSystem1527 : Subsystem  TreatAsAtomicUnit=on  mask(***ATC.DSRVEC***){bd_version=1.01.A, r0=DSM7, r1=-1, r2=, r3=, r4=, r5=, r6=, r7=, r8=, r9=, c0=, c1=, c2=, c3=, c4=, c5=, c6=, c7=, c8=, c9=}
      mask prompts: bd_version "Version"
      mask help: これはAutoCからのブロックです．編集禁止．
      settings: OpenFcn=warndlg('変更禁止!');
      mask Help: web(ui_blockhelpmng(gcb));
      mask Display: disp('ATC.DSRVEC')
  SubSystem1528 : Subsystem  TreatAsAtomicUnit=on  mask(***ATC.DSRVEC***){bd_version=1.01.A, r0=DSM6, r1=-1, r2=, r3=, r4=, r5=, r6=, r7=, r8=, r9=, c0=, c1=, c2=, c3=, c4=, c5=, c6=, c7=, c8=, c9=}
      mask prompts: bd_version "Version"
      mask help: これはAutoCからのブロックです．編集禁止．
      settings: OpenFcn=warndlg('変更禁止!');
      mask Help: web(ui_blockhelpmng(gcb));
      mask Display: disp('ATC.DSRVEC')
  SubSystem1529 : Subsystem  TreatAsAtomicUnit=on  mask(***ATC.DSRVEC***){bd_version=1.01.A, r0=DSM19, r1=-1, r2=, r3=, r4=, r5=, r6=, r7=, r8=, r9=, c0=, c1=, c2=, c3=, c4=, c5=, c6=, c7=, c8=, c9=}
      mask prompts: bd_version "Version"
      mask help: これはAutoCからのブロックです．編集禁止．
      settings: OpenFcn=warndlg('変更禁止!');
      mask Help: web(ui_blockhelpmng(gcb));
      mask Display: disp('ATC.DSRVEC')
  Block4122 : Outport
  wires:
  Block4119/1 -> Block4121/1
  Block4121/1 -> Block4122/1
  SubSystem1527/1(Output) -> Block4121/2
  SubSystem1528/1(Output) -> Block4121/3
  SubSystem1529/1(Output) -> Block4121/4
  Block4120/1 -> SubSystem1527/1(element), SubSystem1528/1(element), SubSystem1529/1(element)

== system lib_all/SubSystem1487/SubSystem1496/SubSystem1530/SubSystem1531 ==
  note: ***編集禁止***
  element : Inport
  Inside_DSR : DataStoreRead  DataStoreName=DSM3
  Selector : Selector  IndexOptions=Index vector (port)  Indices=[1 3]  OutputSizes=1
  Output : Outport
  wires:
  Selector/1 -> Output/1
  element/1 -> Selector/2
  Inside_DSR/1 -> Selector/1

== system lib_all/SubSystem1487/SubSystem1496/SubSystem1530/SubSystem1532 ==
  Block4130 : Inport
  Block4131 : Inport  Port=2
  Block4132 : MultiPortSwitch  zeroidx=off  RndMeth=Zero  SaturateOnIntegerOverflow=on  InputSameDT=on
  SubSystem1533 : Subsystem  TreatAsAtomicUnit=on  mask(***ATC.DSRVEC***){bd_version=1.01.A, r0=DSM7, r1=-1, r2=, r3=, r4=, r5=, r6=, r7=, r8=, r9=, c0=, c1=, c2=, c3=, c4=, c5=, c6=, c7=, c8=, c9=}
      mask prompts: bd_version "Version"
      mask help: これはAutoCからのブロックです．編集禁止．
      settings: OpenFcn=warndlg('変更禁止!');
      mask Help: web(ui_blockhelpmng(gcb));
      mask Display: disp('ATC.DSRVEC')
  SubSystem1534 : Subsystem  TreatAsAtomicUnit=on  mask(***ATC.DSRVEC***){bd_version=1.01.A, r0=DSM6, r1=-1, r2=, r3=, r4=, r5=, r6=, r7=, r8=, r9=, c0=, c1=, c2=, c3=, c4=, c5=, c6=, c7=, c8=, c9=}
      mask prompts: bd_version "Version"
      mask help: これはAutoCからのブロックです．編集禁止．
      settings: OpenFcn=warndlg('変更禁止!');
      mask Help: web(ui_blockhelpmng(gcb));
      mask Display: disp('ATC.DSRVEC')
  SubSystem1535 : Subsystem  TreatAsAtomicUnit=on  mask(***ATC.DSRVEC***){bd_version=1.01.A, r0=DSM19, r1=-1, r2=, r3=, r4=, r5=, r6=, r7=, r8=, r9=, c0=, c1=, c2=, c3=, c4=, c5=, c6=, c7=, c8=, c9=}
      mask prompts: bd_version "Version"
      mask help: これはAutoCからのブロックです．編集禁止．
      settings: OpenFcn=warndlg('変更禁止!');
      mask Help: web(ui_blockhelpmng(gcb));
      mask Display: disp('ATC.DSRVEC')
  Block4133 : Outport
  wires:
  Block4130/1 -> Block4132/1
  Block4132/1 -> Block4133/1
  SubSystem1533/1(Output) -> Block4132/2
  SubSystem1534/1(Output) -> Block4132/3
  SubSystem1535/1(Output) -> Block4132/4
  Block4131/1 -> SubSystem1533/1(element), SubSystem1534/1(element), SubSystem1535/1(element)

== system lib_all/SubSystem1487/SubSystem1496/SubSystem1530/SubSystem1536 ==
  Block4134 : Inport
  Block4135 : Inport  Port=2
  Block4136 : MultiPortSwitch  zeroidx=off  RndMeth=Zero  SaturateOnIntegerOverflow=on  InputSameDT=on
  SubSystem1537 : Subsystem  TreatAsAtomicUnit=on  mask(***ATC.DSRVEC***){bd_version=1.01.A, r0=DSM7, r1=-1, r2=, r3=, r4=, r5=, r6=, r7=, r8=, r9=, c0=, c1=, c2=, c3=, c4=, c5=, c6=, c7=, c8=, c9=}
      mask prompts: bd_version "Version"
      mask help: これはAutoCからのブロックです．編集禁止．
      settings: OpenFcn=warndlg('変更禁止!');
      mask Help: web(ui_blockhelpmng(gcb));
      mask Display: disp('ATC.DSRVEC')
  SubSystem1538 : Subsystem  TreatAsAtomicUnit=on  mask(***ATC.DSRVEC***){bd_version=1.01.A, r0=DSM6, r1=-1, r2=, r3=, r4=, r5=, r6=, r7=, r8=, r9=, c0=, c1=, c2=, c3=, c4=, c5=, c6=, c7=, c8=, c9=}
      mask prompts: bd_version "Version"
      mask help: これはAutoCからのブロックです．編集禁止．
      settings: OpenFcn=warndlg('変更禁止!');
      mask Help: web(ui_blockhelpmng(gcb));
      mask Display: disp('ATC.DSRVEC')
  SubSystem1539 : Subsystem  TreatAsAtomicUnit=on  mask(***ATC.DSRVEC***){bd_version=1.01.A, r0=DSM19, r1=-1, r2=, r3=, r4=, r5=, r6=, r7=, r8=, r9=, c0=, c1=, c2=, c3=, c4=, c5=, c6=, c7=, c8=, c9=}
      mask prompts: bd_version "Version"
      mask help: これはAutoCからのブロックです．編集禁止．
      settings: OpenFcn=warndlg('変更禁止!');
      mask Help: web(ui_blockhelpmng(gcb));
      mask Display: disp('ATC.DSRVEC')
  Block4137 : Outport
  wires:
  Block4134/1 -> Block4136/1
  Block4136/1 -> Block4137/1
  SubSystem1537/1(Output) -> Block4136/2
  SubSystem1538/1(Output) -> Block4136/3
  SubSystem1539/1(Output) -> Block4136/4
  Block4135/1 -> SubSystem1537/1(element), SubSystem1538/1(element), SubSystem1539/1(element)

== system lib_all/SubSystem1540/SubSystem1543/SubSystem1544/Product ==
  note: ***編集禁止***
  mult : Inport
  div : Inport  Port=2
  init : Inport  Port=3
  Constant1 : Constant  OutDataTypeStr=Inherit: Inherit via back propagation
  Constant2 : Constant  Value=0  OutDataTypeStr=Inherit: Inherit via back propagation
  Product : Product  Inputs=*/  SaturateOnIntegerOverflow=on  RndMeth=Zero  InputSameDT=on  OutDataTypeStr=Inherit: Same as first input
  Relational Operator : RelationalOperator  Operator=~=  InputSameDT=on
  Switch : Switch  Threshold=0.5  SaturateOnIntegerOverflow=on  InputSameDT=on
  Switch1 : Switch  Threshold=0.5  SaturateOnIntegerOverflow=on  InputSameDT=on
  Out : Outport
  wires:
  mult/1 -> Product/1
  Product/1 -> Switch/1
  Relational Operator/1 -> Switch/2, Switch1/2
  init/1 -> Switch/3
  Switch/1 -> Out/1
  div/1 -> Switch1/1, Relational Operator/1
  Switch1/1 -> Product/2
  Constant2/1 -> Relational Operator/2
  Constant1/1 -> Switch1/3

== system lib_all/SubSystem1540/SubSystem1543/SubSystem1546/SubSystem1547 ==
  note: ***編集禁止***
  In1 : Inport
  In2 : Inport  Port=2
  RO1 : RelationalOperator  Operator=>  InputSameDT=on
  single1 : DataTypeConversion  OutDataTypeStr=single  SaturateOnIntegerOverflow=on  RndMeth=Zero
  single2 : DataTypeConversion  OutDataTypeStr=single  SaturateOnIntegerOverflow=on  RndMeth=Zero
  Out1 : Outport
  wires:
  single2/1 -> RO1/2
  single1/1 -> RO1/1
  In1/1 -> single1/1
  In2/1 -> single2/1
  RO1/1 -> Out1/1

== system lib_all/SubSystem1540/SubSystem1543/SubSystem1546/SubSystem1548 ==
  note: ***編集禁止***
  In1 : Inport
  In2 : Inport  Port=2
  LO1 : Logic
  RO1 : RelationalOperator  Operator=<  InputSameDT=on
  RO2 : RelationalOperator  Operator=~=  InputSameDT=on
  single1 : DataTypeConversion  OutDataTypeStr=single  SaturateOnIntegerOverflow=on  RndMeth=Zero
  single2 : DataTypeConversion  OutDataTypeStr=single  SaturateOnIntegerOverflow=on  RndMeth=Zero
  Out1 : Outport
  wires:
  LO1/1 -> Out1/1
  RO2/1 -> LO1/2
  RO1/1 -> LO1/1
  single2/1 -> RO2/2, RO1/2
  single1/1 -> RO2/1, RO1/1
  In1/1 -> single1/1
  In2/1 -> single2/1
